simulink model "Cruise_creator"  (R2022a)
settings: Solver=FixedStepAuto  StartTime=0.0  StopTime=10  FixedStep=1e-3  RelTol=1e-3
values from neighbouring files (symbols this model uses):
  base = Mb*9.8/4+mw*9.8+0.5*9.8*Mv    (= 46305)    [parameterSetting.m]
  d = 2.6    [parameterSetting.m]
  I = 3055e-8    (= 3.055e-05)    [parameterSetting.m]
  Jv = 3044    [parameterSetting.m]
  kf = 6.67e7    (= 66700000)    [parameterSetting.m]
  Kv = 1.5e6    (= 1500000)    [parameterSetting.m]
  L = beamLength(k)*210    [BuildDataSample.m]    (also in parameterSetting.m = 210)
  Ln = length_set(i)    [DatacreatorForChapter4.m]
  Ln2 = 0    [DatacreatorForChapter4.m]
  m = 7800*(7684e-6)    (= 59.9352)    [parameterSetting.m]
  Mv = 4060/2    (= 2030)    [parameterSetting.m]
  mw = 1670/2    (= 835)    [parameterSetting.m]
  position = 0.55*L    [DatacreatorForChapter4.m]    (also in Figure4_9.m = 94.5; chapter4_3.m = 105; parameterSetting.m = 105)
  R = 0.42    [parameterSetting.m]
  r = 0.25    [chapter4_3.m]    (also in parameterSetting.m = 0.42)
  v = vkmh/3.6    [BuildDataSample.m]    (also in DatacreatorForChapter4.m = vkmh_set(j)/3.6; Figure4_9.m = vkmh_set(i); chapter4_1.m = vkmh_set(i)/3.6; chapter4_3.m = 13.8888888889)
  vkmh = vkmh_set(j)    [BuildDataSample.m]    (also in parameterSetting.m = 100)
(part 1 of 79)
settings Model: Creator=Spicydel  LastModifiedBy=Spicydel

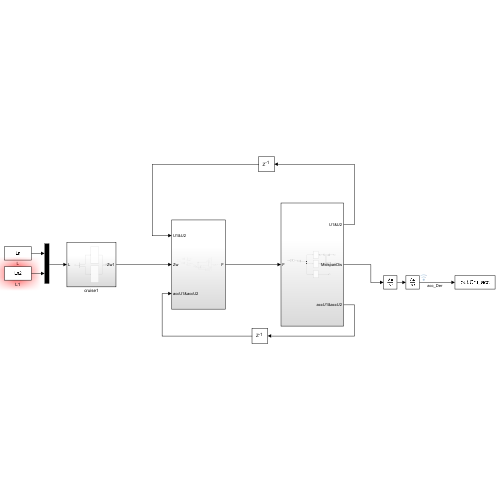
[thumbnail: Simulink's stored preview of the top-level diagram]
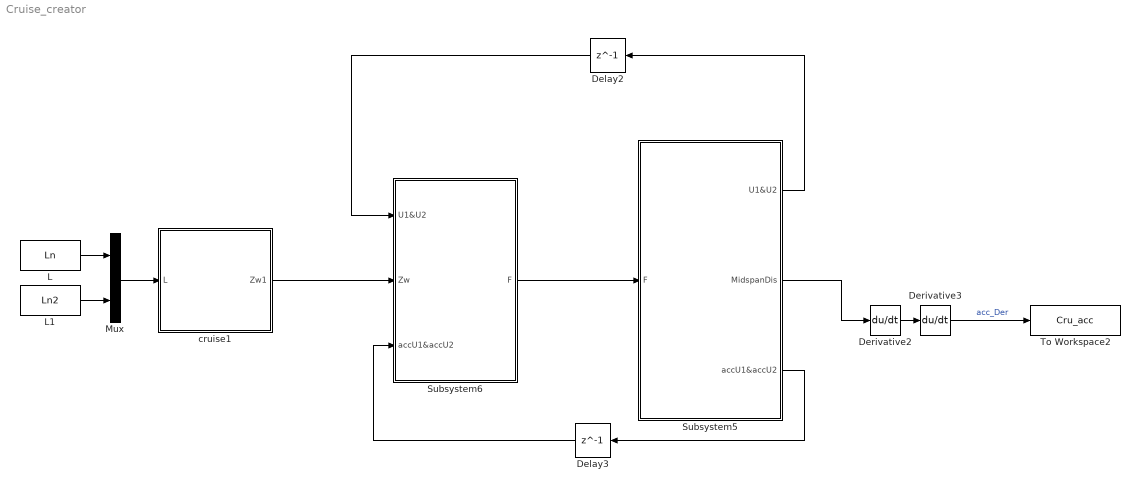
== system Cruise_creator ==
  Delay2 : Delay  DelayLength=1
  Delay3 : Delay  DelayLength=1
  Derivative2 : Derivative
  Derivative3 : Derivative
  L : Constant  Value=Ln
  L1 : Constant  Value=Ln2
  Mux : Mux  Inputs=2
  Subsystem5 : Subsystem
  Subsystem6 : Subsystem
  To Workspace2 : ToWorkspace  VariableName=Cru_acc  SaveFormat=Timeseries  SampleTime=-1
  cruise1 : Subsystem
  wires:
  Mux/1 -> cruise1/1(L)
  L1/1 -> Mux/2
  L/1 -> Mux/1
  Subsystem5/3(accU1&accU2) -> Delay3/1
  Derivative3/1 -> To Workspace2/1  "acc_Der"
  Derivative2/1 -> Derivative3/1
  Subsystem5/2(MidspanDis) -> Derivative2/1
  Delay2/1 -> Subsystem6/1(U1&U2)
  Subsystem5/1(U1&U2) -> Delay2/1
  Subsystem6/1(F) -> Subsystem5/1(F)
  cruise1/1(Zw1) -> Subsystem6/2(Zw)
  Delay3/1 -> Subsystem6/3(accU1&accU2)

== system Cruise_creator/Subsystem5 ==
  F : Inport
  Add : Sum
  Demux1 : Demux  Outputs=2
  Demux2 : Demux  Outputs=2
  Demux3 : Demux  Outputs=2
  Gain1 : Gain  Gain=-1
  Gain2 : Gain  Gain=-1
  Mux : Mux  Inputs=2
  Mux1 : Mux  Inputs=2
  Mux2 : Mux  Inputs=2
  Subsystem : Subsystem
  Subsystem1 : Subsystem
  Subsystem3 : Subsystem
  U1&U2 : Outport
  MidspanDis : Outport  Port=2
  accU1&accU2 : Outport  Port=3
  wires:
  Demux3/2 -> Mux2/2
  Demux2/2 -> Mux2/1
  Mux/1 -> U1&U2/1
  Mux2/1 -> accU1&accU2/1
  Demux3/1 -> Mux/2
  Demux2/1 -> Mux/1
  Gain2/1 -> Demux3/1
  Mux1/1 -> Subsystem/1(F), Subsystem1/1(F), Subsystem3/1(F)
  Add/1 -> Mux1/1, Mux1/2
  Demux1/2 -> Add/2
  Demux1/1 -> Add/1
  F/1 -> Demux1/1
  Gain1/1 -> Demux2/1
  Subsystem1/1(u2) -> Gain2/1
  Subsystem/1(u1) -> Gain1/1
  Subsystem3/1(Midspan) -> MidspanDis/1

== system Cruise_creator/Subsystem6 ==
  U1&U2 : Inport
  Zw : Inport  Port=2
  accU1&accU2 : Inport  Port=3
  Add : Sum  Inputs=+---
  Add1 : Sum  Inputs=-+
  Add2 : Sum  Inputs=--++-
  Add3 : Sum  Inputs=-++--
  Add4 : Sum
  Add5 : Sum  Inputs=-+
  Add6 : Sum  Inputs=-+
  Add7 : Sum  Inputs=++--
  Constant : Constant  Value=base
  Constant1 : Constant  Value=0
  Demux{,1..6} (7 alike) : Demux  Outputs=2
  Derivative{,1..3} (4 alike) : Derivative
  Gain : Gain  Gain=2*Kv/Mv
  Gain1 : Gain  Gain=Kv*d*d/Jv/2
  Gain12 : Gain  Gain=Kv/Mv
  Gain14 : Gain  Gain=Kv*d/Jv/2
  Gain2 : Gain  Gain=Kv
  Gain3 : Gain  Gain=Kv/Mv
  Gain4 : Gain  Gain=Kv*d/Jv/2
  Gain5 : Gain  Gain=d*Kv/2
  Gain7 : Gain  Gain=mw
  Gain8 : Gain  Gain=mw
  Gain9 : Gain  Gain=Kv
  Integrator{,1..3} (4 alike) : Integrator
  Max : MinMax  Function=max  Inputs=2
  Mux : Mux  Inputs=2
  F : Outport
  wires:
  Add/1 -> Mux/1  "F1"
  Add7/1 -> Mux/2  "F2"
  Gain8/1 -> Add7/4
  Demux4/2 -> Add7/3
  Gain9/1 -> Demux4/1
  Gain7/1 -> Add/4
  Add6/1 -> Gain8/1
  Add5/1 -> Gain7/1
  Demux4/1 -> Add/3
  Demux5/2 -> Add6/1
  Derivative3/1 -> Add6/2
  Demux5/1 -> Add5/1
  Derivative1/1 -> Add5/2
  Demux6/2 -> Derivative2/1
  Derivative2/1 -> Derivative3/1
  Demux6/1 -> Derivative/1
  accU1&accU2/1 -> Demux5/1
  Derivative/1 -> Derivative1/1
  Add1/1 -> Gain9/1
  [Zw] -> Add1/2, Demux6/1
  [U1u2] -> Add1/1
  [Zw] -> Gain4/1
  Gain4/1 -> Demux2/1
  Gain3/1 -> Demux/1
  [Zw] -> Gain3/1
  Gain2/1 -> Add/1, Add7/1
  Demux3/2 -> Add3/4
  Demux3/1 -> Add3/3
  Gain14/1 -> Demux3/1
  [U1u2] -> Gain14/1
  Demux1/1 -> Add2/1
  Demux1/2 -> Add2/2
  Gain12/1 -> Demux1/1
  [U1u2] -> Gain12/1
  U1&U2/1 -> [U1u2]
  Demux2/2 -> Add3/2
  Demux2/1 -> Add3/1
  Gain1/1 -> Add3/5  "theta_dot_dot"
  Demux/2 -> Add2/4
  Demux/1 -> Add2/3
  Gain/1 -> Add2/5  "Z_dot_dot"
  Zw/1 -> [Zw]
  Gain5/1 -> Add7/2, Add/2
  Add3/1 -> Integrator2/1  "theta_dot_dot"
  Integrator3/1 -> Gain1/1, Gain5/1  "theta"
  Integrator2/1 -> Integrator3/1
  Add2/1 -> Integrator/1  "Z_dot_dot"
  Integrator1/1 -> Gain/1, Gain2/1  "Zv"
  Integrator/1 -> Integrator1/1
  Constant1/1 -> Max/2
  Add4/1 -> Max/1
  Mux/1 -> Add4/1
  Constant/1 -> Add4/2
  Max/1 -> F/1

== system Cruise_creator/cruise1 ==
  L : Inport
  Demux : Demux  Outputs=2
  Mux : Mux  Inputs=2
  Subsystem1 : Subsystem
  Subsystem2 : Subsystem
  Zw1 : Outport
  wires:
  L/1 -> Demux/1
  Demux/1 -> Subsystem1/1(L0)
  Demux/2 -> Subsystem2/1(L0)
  Subsystem1/1(Output) -> Mux/1
  Subsystem2/1(Output) -> Mux/2
  Mux/1 -> Zw1/1

== system Cruise_creator/Subsystem5/Subsystem ==
  F : Inport
  Add : Sum  Inputs=+++++++
  Constant1 : Constant  Value=21
  Constant11 : Constant
  Constant2 : Constant  Value=41
  Constant3 : Constant  Value=61
  Constant4 : Constant  Value=81
  Constant5 : Constant  Value=101
  Constant6 : Constant  Value=121
  Subsystem : Subsystem
  Subsystem1 : Subsystem
  Subsystem2 : Subsystem
  Subsystem3 : Subsystem
  Subsystem4 : Subsystem
  Subsystem5 : Subsystem
  Subsystem6 : Subsystem
  u1 : Outport
  wires:
  Subsystem6/1(Out1) -> Add/7
  [Ff] -> Subsystem6/2(In2)
  Constant6/1 -> Subsystem6/1(In1)
  Subsystem5/1(Out1) -> Add/6
  Constant5/1 -> Subsystem5/1(In1)
  [Ff] -> Subsystem5/2(In2)
  Add/1 -> u1/1
  Subsystem4/1(Out1) -> Add/5
  Subsystem3/1(Out1) -> Add/4
  Subsystem2/1(Out1) -> Add/3
  Subsystem1/1(Out1) -> Add/2
  Subsystem/1(Out1) -> Add/1
  [Ff] -> Subsystem4/2(In2)
  Constant4/1 -> Subsystem4/1(In1)
  Constant3/1 -> Subsystem3/1(In1)
  [Ff] -> Subsystem3/2(In2)
  [Ff] -> Subsystem2/2(In2)
  Constant2/1 -> Subsystem2/1(In1)
  Constant1/1 -> Subsystem1/1(In1)
  [Ff] -> Subsystem1/2(In2)
  [Ff] -> Subsystem/2(In2)
  Constant11/1 -> Subsystem/1(In1)
  F/1 -> [Ff]

== system Cruise_creator/Subsystem5/Subsystem1 ==
  F : Inport
  Add : Sum  Inputs=+++++++
  Constant1 : Constant  Value=21
  Constant11 : Constant
  Constant2 : Constant  Value=61
  Constant3 : Constant  Value=41
  Constant4 : Constant  Value=101
  Constant5 : Constant  Value=81
  Constant6 : Constant  Value=121
  Subsystem : Subsystem
  Subsystem1 : Subsystem
  Subsystem2 : Subsystem
  Subsystem3 : Subsystem
  Subsystem4 : Subsystem
  Subsystem5 : Subsystem
  Subsystem6 : Subsystem
  u2 : Outport
  wires:
  Subsystem6/1(Out1) -> Add/7
  Subsystem5/1(Out1) -> Add/6
  Constant4/1 -> Subsystem5/1(In1)
  [Ff] -> Subsystem5/2(In2)
  Constant6/1 -> Subsystem6/1(In1)
  [Ff] -> Subsystem6/2(In2)
  Add/1 -> u2/1
  Subsystem4/1(Out1) -> Add/5
  Subsystem3/1(Out1) -> Add/4
  Subsystem2/1(Out1) -> Add/3
  Subsystem1/1(Out1) -> Add/2
  [Ff] -> Subsystem4/2(In2)
  Constant5/1 -> Subsystem4/1(In1)
  Constant3/1 -> Subsystem2/1(In1)
  [Ff] -> Subsystem2/2(In2)
  [Ff] -> Subsystem3/2(In2)
  Constant2/1 -> Subsystem3/1(In1)
  Constant1/1 -> Subsystem1/1(In1)
  [Ff] -> Subsystem1/2(In2)
  [Ff] -> Subsystem/2(In2)
  Subsystem/1(Out1) -> Add/1
  Constant11/1 -> Subsystem/1(In1)
  F/1 -> [Ff]

== system Cruise_creator/Subsystem5/Subsystem3 ==
  F : Inport
  Add : Sum  Inputs=+++++++
  Constant1 : Constant  Value=21
  Constant11 : Constant
  Constant2 : Constant  Value=41
  Constant3 : Constant  Value=61
  Constant4 : Constant  Value=81
  Constant5 : Constant  Value=101
  Constant6 : Constant  Value=121
  Subsystem1 : Subsystem
  Subsystem2 : Subsystem
  Subsystem3 : Subsystem
  Subsystem4 : Subsystem
  Subsystem5 : Subsystem
  Subsystem6 : Subsystem
  Subsystem7 : Subsystem
  Midspan : Outport
  wires:
  Subsystem7/1(Out1) -> Add/7
  Subsystem6/1(Out1) -> Add/6
  [Ff] -> Subsystem7/1(In2)
  Constant6/1 -> Subsystem7/2(In1)
  Constant5/1 -> Subsystem6/2(In1)
  [Ff] -> Subsystem6/1(In2)
  Subsystem4/1(Out1) -> Add/5
  Subsystem3/1(Out1) -> Add/4
  [Ff] -> Subsystem4/1(In2)
  Constant4/1 -> Subsystem4/2(In1)
  Constant3/1 -> Subsystem3/2(In1)
  [Ff] -> Subsystem3/1(In2)
  [Ff] -> Subsystem2/1(In2)
  [Ff] -> Subsystem1/1(In2)
  [Ff] -> Subsystem5/1(In2)
  Add/1 -> Midspan/1
  Subsystem2/1(Out1) -> Add/3
  Constant2/1 -> Subsystem2/2(In1)
  Subsystem1/1(Out1) -> Add/2
  Constant1/1 -> Subsystem1/2(In1)
  Subsystem5/1(Out1) -> Add/1
  Constant11/1 -> Subsystem5/2(In1)
  F/1 -> [Ff]

== system Cruise_creator/cruise1/Subsystem1 ==
  L0 : Inport
  Constant : Constant  Value=0
  Scope : Scope
  Subsystem : Subsystem
  Switch : Switch  Criteria=u2 ~= 0  SaturateOnIntegerOverflow=off  InputSameDT=off
  Output : Outport
  wires:
  L0/1 -> Subsystem/1(L), Subsystem/2(L0), Switch/2
  Switch/1 -> Output/1
  Constant/1 -> Switch/3
  Subsystem/1(Output) -> Scope/1, Switch/1

== system Cruise_creator/cruise1/Subsystem2 ==
  L0 : Inport
  Constant : Constant  Value=0
  Subsystem : Subsystem
  Switch : Switch  Criteria=u2 ~= 0  SaturateOnIntegerOverflow=off  InputSameDT=off
  Output : Outport
  wires:
  Subsystem/1(Output) -> Switch/1
  Constant/1 -> Switch/3
  Switch/1 -> Output/1
  L0/1 -> Subsystem/2(L0), Switch/2, Subsystem/1(L)

== system Cruise_creator/Subsystem5/Subsystem/Subsystem ==
  In1 : Inport
  In2 : Inport  Port=2
  Add : Sum  Inputs=++++++++++++++++++++
  Add Constant{,1..18} (19 alike) : Bias  Bias=1  SaturateOnIntegerOverflow=off
  Subsystem1 : Subsystem
  Subsystem10 : Subsystem
  Subsystem11 : Subsystem
  Subsystem12 : Subsystem
  Subsystem13 : Subsystem
  Subsystem14 : Subsystem
  Subsystem15 : Subsystem
  Subsystem16 : Subsystem
  Subsystem17 : Subsystem
  Subsystem18 : Subsystem
  Subsystem19 : Subsystem
  Subsystem2 : Subsystem
  Subsystem20 : Subsystem
  Subsystem3 : Subsystem
  Subsystem4 : Subsystem
  Subsystem5 : Subsystem
  Subsystem6 : Subsystem
  Subsystem7 : Subsystem
  Subsystem8 : Subsystem
  Subsystem9 : Subsystem
  Out1 : Outport
  wires:
  In2/1 -> [Ff]
  Add/1 -> Out1/1
  [Ff] -> Subsystem18/2(F)
  [Ff] -> Subsystem16/2(F)
  Add Constant7/1 -> Add Constant8/1, Subsystem14/1(index)
  Add Constant4/1 -> Add Constant5/1, Subsystem4/1(index)
  Add Constant6/1 -> Add Constant7/1, Subsystem5/1(index)
  Add Constant5/1 -> Add Constant6/1, Subsystem7/1(index)
  Subsystem11/1(Out1) -> Add/11
  Subsystem18/1(Out1) -> Add/20
  [Ff] -> Subsystem12/2(F)
  [Ff] -> Subsystem14/2(F)
  [Ff] -> Subsystem5/2(F)
  [Ff] -> Subsystem9/2(F)
  [Ff] -> Subsystem4/2(F)
  Subsystem4/1(Out1) -> Add/6
  Add Constant12/1 -> Add Constant13/1, Subsystem12/1(index)
  Subsystem2/1(Out1) -> Add/4
  Add Constant13/1 -> Add Constant14/1, Subsystem15/1(index)
  Subsystem15/1(Out1) -> Add/15
  [Ff] -> Subsystem11/2(F)
  [Ff] -> Subsystem7/2(F)
  [Ff] -> Subsystem6/2(F)
  Subsystem13/1(Out1) -> Add/16
  Subsystem16/1(Out1) -> Add/13
  In1/1 -> Add Constant/1, Subsystem6/1(index)
  Subsystem12/1(Out1) -> Add/14
  Subsystem20/1(Out1) -> Add/17
  [Ff] -> Subsystem8/2(F)
  Subsystem3/1(Out1) -> Add/3
  [Ff] -> Subsystem2/2(F)
  Add Constant8/1 -> Add Constant9/1, Subsystem9/1(index)
  [Ff] -> Subsystem19/2(F)
  [Ff] -> Subsystem1/2(F)
  Subsystem19/1(Out1) -> Add/19
  Subsystem17/1(Out1) -> Add/18
  [Ff] -> Subsystem20/2(F)
  Add Constant10/1 -> Add Constant11/1, Subsystem10/1(index)
  Add Constant16/1 -> Add Constant17/1, Subsystem17/1(index)
  Subsystem6/1(Out1) -> Add/1
  Subsystem10/1(Out1) -> Add/12
  Subsystem5/1(Out1) -> Add/8
  [Ff] -> Subsystem10/2(F)
  [Ff] -> Subsystem15/2(F)
  Add Constant18/1 -> Subsystem18/1(index)
  Add Constant17/1 -> Add Constant18/1, Subsystem19/1(index)
  Subsystem7/1(Out1) -> Add/7
  Add Constant1/1 -> Add Constant2/1, Subsystem3/1(index)
  [Ff] -> Subsystem3/2(F)
  Subsystem9/1(Out1) -> Add/10
  Add Constant3/1 -> Add Constant4/1, Subsystem8/1(index)
  Add Constant/1 -> Add Constant1/1, Subsystem1/1(index)
  Subsystem1/1(Out1) -> Add/2
  Add Constant15/1 -> Add Constant16/1, Subsystem20/1(index)
  [Ff] -> Subsystem17/2(F)
  Subsystem8/1(Out1) -> Add/5
  Add Constant14/1 -> Add Constant15/1, Subsystem13/1(index)
  Add Constant11/1 -> Add Constant12/1, Subsystem16/1(index)
  [Ff] -> Subsystem13/2(F)
  Add Constant2/1 -> Add Constant3/1, Subsystem2/1(index)
  Add Constant9/1 -> Add Constant10/1, Subsystem11/1(index)
  Subsystem14/1(Out1) -> Add/9

== system Cruise_creator/Subsystem5/Subsystem/Subsystem1 ==
  In1 : Inport
  In2 : Inport  Port=2
  Add : Sum  Inputs=++++++++++++++++++++
  Add Constant{,1..18} (19 alike) : Bias  Bias=1  SaturateOnIntegerOverflow=off
  Subsystem1 : Subsystem
  Subsystem10 : Subsystem
  Subsystem11 : Subsystem
  Subsystem12 : Subsystem
  Subsystem13 : Subsystem
  Subsystem14 : Subsystem
  Subsystem15 : Subsystem
  Subsystem16 : Subsystem
  Subsystem17 : Subsystem
  Subsystem18 : Subsystem
  Subsystem19 : Subsystem
  Subsystem2 : Subsystem
  Subsystem20 : Subsystem
  Subsystem3 : Subsystem
  Subsystem4 : Subsystem
  Subsystem5 : Subsystem
  Subsystem6 : Subsystem
  Subsystem7 : Subsystem
  Subsystem8 : Subsystem
  Subsystem9 : Subsystem
  Out1 : Outport
  wires:
  Subsystem14/1(Out1) -> Add/9
  Add Constant9/1 -> Subsystem11/1(index), Add Constant10/1
  Add Constant2/1 -> Subsystem2/1(index), Add Constant3/1
  [Ff] -> Subsystem13/2(F)
  Add Constant11/1 -> Subsystem16/1(index), Add Constant12/1
  Add Constant14/1 -> Subsystem13/1(index), Add Constant15/1
  Subsystem8/1(Out1) -> Add/5
  [Ff] -> Subsystem17/2(F)
  Add Constant15/1 -> Subsystem20/1(index), Add Constant16/1
  Subsystem1/1(Out1) -> Add/2
  Add Constant/1 -> Subsystem1/1(index), Add Constant1/1
  Add Constant3/1 -> Subsystem8/1(index), Add Constant4/1
  Subsystem9/1(Out1) -> Add/10
  [Ff] -> Subsystem3/2(F)
  Add Constant1/1 -> Subsystem3/1(index), Add Constant2/1
  Subsystem7/1(Out1) -> Add/7
  Add Constant17/1 -> Subsystem19/1(index), Add Constant18/1
  Add Constant18/1 -> Subsystem18/1(index)
  [Ff] -> Subsystem15/2(F)
  [Ff] -> Subsystem10/2(F)
  Subsystem5/1(Out1) -> Add/8
  Subsystem10/1(Out1) -> Add/12
  Subsystem6/1(Out1) -> Add/1
  Add Constant16/1 -> Subsystem17/1(index), Add Constant17/1
  Add Constant10/1 -> Subsystem10/1(index), Add Constant11/1
  [Ff] -> Subsystem20/2(F)
  Subsystem17/1(Out1) -> Add/18
  Subsystem19/1(Out1) -> Add/19
  [Ff] -> Subsystem1/2(F)
  [Ff] -> Subsystem19/2(F)
  Add Constant8/1 -> Subsystem9/1(index), Add Constant9/1
  [Ff] -> Subsystem2/2(F)
  Subsystem3/1(Out1) -> Add/3
  [Ff] -> Subsystem8/2(F)
  Subsystem20/1(Out1) -> Add/17
  Subsystem12/1(Out1) -> Add/14
  In1/1 -> Subsystem6/1(index), Add Constant/1
  Subsystem16/1(Out1) -> Add/13
  Subsystem13/1(Out1) -> Add/16
  [Ff] -> Subsystem6/2(F)
  [Ff] -> Subsystem7/2(F)
  [Ff] -> Subsystem11/2(F)
  Subsystem15/1(Out1) -> Add/15
  Add Constant13/1 -> Subsystem15/1(index), Add Constant14/1
  Subsystem2/1(Out1) -> Add/4
  Add Constant12/1 -> Subsystem12/1(index), Add Constant13/1
  Subsystem4/1(Out1) -> Add/6
  [Ff] -> Subsystem4/2(F)
  [Ff] -> Subsystem9/2(F)
  [Ff] -> Subsystem5/2(F)
  [Ff] -> Subsystem14/2(F)
  [Ff] -> Subsystem12/2(F)
  Subsystem18/1(Out1) -> Add/20
  Subsystem11/1(Out1) -> Add/11
  Add Constant5/1 -> Subsystem7/1(index), Add Constant6/1
  Add Constant6/1 -> Subsystem5/1(index), Add Constant7/1
  Add Constant4/1 -> Subsystem4/1(index), Add Constant5/1
  Add Constant7/1 -> Subsystem14/1(index), Add Constant8/1
  [Ff] -> Subsystem16/2(F)
  [Ff] -> Subsystem18/2(F)
  Add/1 -> Out1/1
  In2/1 -> [Ff]

== system Cruise_creator/Subsystem5/Subsystem/Subsystem2 ==
  In1 : Inport
  In2 : Inport  Port=2
  Add : Sum  Inputs=++++++++++++++++++++
  Add Constant{,1..18} (19 alike) : Bias  Bias=1  SaturateOnIntegerOverflow=off
  Subsystem1 : Subsystem
  Subsystem10 : Subsystem
  Subsystem11 : Subsystem
  Subsystem12 : Subsystem
  Subsystem13 : Subsystem
  Subsystem14 : Subsystem
  Subsystem15 : Subsystem
  Subsystem16 : Subsystem
  Subsystem17 : Subsystem
  Subsystem18 : Subsystem
  Subsystem19 : Subsystem
  Subsystem2 : Subsystem
  Subsystem20 : Subsystem
  Subsystem3 : Subsystem
  Subsystem4 : Subsystem
  Subsystem5 : Subsystem
  Subsystem6 : Subsystem
  Subsystem7 : Subsystem
  Subsystem8 : Subsystem
  Subsystem9 : Subsystem
  Out1 : Outport
  wires:
  In2/1 -> [Ff]
  Add/1 -> Out1/1
  [Ff] -> Subsystem18/2(F)
  [Ff] -> Subsystem16/2(F)
  Add Constant7/1 -> Add Constant8/1, Subsystem14/1(index)
  Add Constant4/1 -> Add Constant5/1, Subsystem4/1(index)
  Add Constant6/1 -> Add Constant7/1, Subsystem5/1(index)
  Add Constant5/1 -> Add Constant6/1, Subsystem7/1(index)
  Subsystem11/1(Out1) -> Add/11
  Subsystem18/1(Out1) -> Add/20
  [Ff] -> Subsystem12/2(F)
  [Ff] -> Subsystem14/2(F)
  [Ff] -> Subsystem5/2(F)
  [Ff] -> Subsystem9/2(F)
  [Ff] -> Subsystem4/2(F)
  Subsystem4/1(Out1) -> Add/6
  Add Constant12/1 -> Add Constant13/1, Subsystem12/1(index)
  Subsystem2/1(Out1) -> Add/4
  Add Constant13/1 -> Add Constant14/1, Subsystem15/1(index)
  Subsystem15/1(Out1) -> Add/15
  [Ff] -> Subsystem11/2(F)
  [Ff] -> Subsystem7/2(F)
  [Ff] -> Subsystem6/2(F)
  Subsystem13/1(Out1) -> Add/16
  Subsystem16/1(Out1) -> Add/13
  In1/1 -> Add Constant/1, Subsystem6/1(index)
  Subsystem12/1(Out1) -> Add/14
  Subsystem20/1(Out1) -> Add/17
  [Ff] -> Subsystem8/2(F)
  Subsystem3/1(Out1) -> Add/3
  [Ff] -> Subsystem2/2(F)
  Add Constant8/1 -> Add Constant9/1, Subsystem9/1(index)
  [Ff] -> Subsystem19/2(F)
  [Ff] -> Subsystem1/2(F)
  Subsystem19/1(Out1) -> Add/19
  Subsystem17/1(Out1) -> Add/18
  [Ff] -> Subsystem20/2(F)
  Add Constant10/1 -> Add Constant11/1, Subsystem10/1(index)
  Add Constant16/1 -> Add Constant17/1, Subsystem17/1(index)
  Subsystem6/1(Out1) -> Add/1
  Subsystem10/1(Out1) -> Add/12
  Subsystem5/1(Out1) -> Add/8
  [Ff] -> Subsystem10/2(F)
  [Ff] -> Subsystem15/2(F)
  Add Constant18/1 -> Subsystem18/1(index)
  Add Constant17/1 -> Add Constant18/1, Subsystem19/1(index)
  Subsystem7/1(Out1) -> Add/7
  Add Constant1/1 -> Add Constant2/1, Subsystem3/1(index)
  [Ff] -> Subsystem3/2(F)
  Subsystem9/1(Out1) -> Add/10
  Add Constant3/1 -> Add Constant4/1, Subsystem8/1(index)
  Add Constant/1 -> Add Constant1/1, Subsystem1/1(index)
  Subsystem1/1(Out1) -> Add/2
  Add Constant15/1 -> Add Constant16/1, Subsystem20/1(index)
  [Ff] -> Subsystem17/2(F)
  Subsystem8/1(Out1) -> Add/5
  Add Constant14/1 -> Add Constant15/1, Subsystem13/1(index)
  Add Constant11/1 -> Add Constant12/1, Subsystem16/1(index)
  [Ff] -> Subsystem13/2(F)
  Add Constant2/1 -> Add Constant3/1, Subsystem2/1(index)
  Add Constant9/1 -> Add Constant10/1, Subsystem11/1(index)
  Subsystem14/1(Out1) -> Add/9

== system Cruise_creator/Subsystem5/Subsystem/Subsystem3 ==
  In1 : Inport
  In2 : Inport  Port=2
  Add : Sum  Inputs=++++++++++++++++++++
  Add Constant{,1..18} (19 alike) : Bias  Bias=1  SaturateOnIntegerOverflow=off
  Subsystem1 : Subsystem
  Subsystem10 : Subsystem
  Subsystem11 : Subsystem
  Subsystem12 : Subsystem
  Subsystem13 : Subsystem
  Subsystem14 : Subsystem
  Subsystem15 : Subsystem
  Subsystem16 : Subsystem
  Subsystem17 : Subsystem
  Subsystem18 : Subsystem
  Subsystem19 : Subsystem
  Subsystem2 : Subsystem
  Subsystem20 : Subsystem
  Subsystem3 : Subsystem
  Subsystem4 : Subsystem
  Subsystem5 : Subsystem
  Subsystem6 : Subsystem
  Subsystem7 : Subsystem
  Subsystem8 : Subsystem
  Subsystem9 : Subsystem
  Out1 : Outport
  wires:
  Subsystem14/1(Out1) -> Add/9
  Add Constant9/1 -> Subsystem11/1(index), Add Constant10/1
  Add Constant2/1 -> Subsystem2/1(index), Add Constant3/1
  [Ff] -> Subsystem13/2(F)
  Add Constant11/1 -> Subsystem16/1(index), Add Constant12/1
  Add Constant14/1 -> Subsystem13/1(index), Add Constant15/1
  Subsystem8/1(Out1) -> Add/5
  [Ff] -> Subsystem17/2(F)
  Add Constant15/1 -> Subsystem20/1(index), Add Constant16/1
  Subsystem1/1(Out1) -> Add/2
  Add Constant/1 -> Subsystem1/1(index), Add Constant1/1
  Add Constant3/1 -> Subsystem8/1(index), Add Constant4/1
  Subsystem9/1(Out1) -> Add/10
  [Ff] -> Subsystem3/2(F)
  Add Constant1/1 -> Subsystem3/1(index), Add Constant2/1
  Subsystem7/1(Out1) -> Add/7
  Add Constant17/1 -> Subsystem19/1(index), Add Constant18/1
  Add Constant18/1 -> Subsystem18/1(index)
  [Ff] -> Subsystem15/2(F)
  [Ff] -> Subsystem10/2(F)
  Subsystem5/1(Out1) -> Add/8
  Subsystem10/1(Out1) -> Add/12
  Subsystem6/1(Out1) -> Add/1
  Add Constant16/1 -> Subsystem17/1(index), Add Constant17/1
  Add Constant10/1 -> Subsystem10/1(index), Add Constant11/1
  [Ff] -> Subsystem20/2(F)
  Subsystem17/1(Out1) -> Add/18
  Subsystem19/1(Out1) -> Add/19
  [Ff] -> Subsystem1/2(F)
  [Ff] -> Subsystem19/2(F)
  Add Constant8/1 -> Subsystem9/1(index), Add Constant9/1
  [Ff] -> Subsystem2/2(F)
  Subsystem3/1(Out1) -> Add/3
  [Ff] -> Subsystem8/2(F)
  Subsystem20/1(Out1) -> Add/17
  Subsystem12/1(Out1) -> Add/14
  In1/1 -> Subsystem6/1(index), Add Constant/1
  Subsystem16/1(Out1) -> Add/13
  Subsystem13/1(Out1) -> Add/16
  [Ff] -> Subsystem6/2(F)
  [Ff] -> Subsystem7/2(F)
  [Ff] -> Subsystem11/2(F)
  Subsystem15/1(Out1) -> Add/15
  Add Constant13/1 -> Subsystem15/1(index), Add Constant14/1
  Subsystem2/1(Out1) -> Add/4
  Add Constant12/1 -> Subsystem12/1(index), Add Constant13/1
  Subsystem4/1(Out1) -> Add/6
  [Ff] -> Subsystem4/2(F)
  [Ff] -> Subsystem9/2(F)
  [Ff] -> Subsystem5/2(F)
  [Ff] -> Subsystem14/2(F)
  [Ff] -> Subsystem12/2(F)
  Subsystem18/1(Out1) -> Add/20
  Subsystem11/1(Out1) -> Add/11
  Add Constant5/1 -> Subsystem7/1(index), Add Constant6/1
  Add Constant6/1 -> Subsystem5/1(index), Add Constant7/1
  Add Constant4/1 -> Subsystem4/1(index), Add Constant5/1
  Add Constant7/1 -> Subsystem14/1(index), Add Constant8/1
  [Ff] -> Subsystem16/2(F)
  [Ff] -> Subsystem18/2(F)
  Add/1 -> Out1/1
  In2/1 -> [Ff]

== system Cruise_creator/Subsystem5/Subsystem/Subsystem4 ==
  In1 : Inport
  In2 : Inport  Port=2
  Add : Sum  Inputs=++++++++++++++++++++
  Add Constant{,1..18} (19 alike) : Bias  Bias=1  SaturateOnIntegerOverflow=off
  Subsystem1 : Subsystem
  Subsystem10 : Subsystem
  Subsystem11 : Subsystem
  Subsystem12 : Subsystem
  Subsystem13 : Subsystem
  Subsystem14 : Subsystem
  Subsystem15 : Subsystem
  Subsystem16 : Subsystem
  Subsystem17 : Subsystem
  Subsystem18 : Subsystem
  Subsystem19 : Subsystem
  Subsystem2 : Subsystem
  Subsystem20 : Subsystem
  Subsystem3 : Subsystem
  Subsystem4 : Subsystem
  Subsystem5 : Subsystem
  Subsystem6 : Subsystem
  Subsystem7 : Subsystem
  Subsystem8 : Subsystem
  Subsystem9 : Subsystem
  Out1 : Outport
  wires:
  In2/1 -> [Ff]
  Add/1 -> Out1/1
  [Ff] -> Subsystem18/2(F)
  [Ff] -> Subsystem16/2(F)
  Add Constant7/1 -> Add Constant8/1, Subsystem14/1(index)
  Add Constant4/1 -> Add Constant5/1, Subsystem4/1(index)
  Add Constant6/1 -> Add Constant7/1, Subsystem5/1(index)
  Add Constant5/1 -> Add Constant6/1, Subsystem7/1(index)
  Subsystem11/1(Out1) -> Add/11
  Subsystem18/1(Out1) -> Add/20
  [Ff] -> Subsystem12/2(F)
  [Ff] -> Subsystem14/2(F)
  [Ff] -> Subsystem5/2(F)
  [Ff] -> Subsystem9/2(F)
  [Ff] -> Subsystem4/2(F)
  Subsystem4/1(Out1) -> Add/6
  Add Constant12/1 -> Add Constant13/1, Subsystem12/1(index)
  Subsystem2/1(Out1) -> Add/4
  Add Constant13/1 -> Add Constant14/1, Subsystem15/1(index)
  Subsystem15/1(Out1) -> Add/15
  [Ff] -> Subsystem11/2(F)
  [Ff] -> Subsystem7/2(F)
  [Ff] -> Subsystem6/2(F)
  Subsystem13/1(Out1) -> Add/16
  Subsystem16/1(Out1) -> Add/13
  In1/1 -> Add Constant/1, Subsystem6/1(index)
  Subsystem12/1(Out1) -> Add/14
  Subsystem20/1(Out1) -> Add/17
  [Ff] -> Subsystem8/2(F)
  Subsystem3/1(Out1) -> Add/3
  [Ff] -> Subsystem2/2(F)
  Add Constant8/1 -> Add Constant9/1, Subsystem9/1(index)
  [Ff] -> Subsystem19/2(F)
  [Ff] -> Subsystem1/2(F)
  Subsystem19/1(Out1) -> Add/19
  Subsystem17/1(Out1) -> Add/18
  [Ff] -> Subsystem20/2(F)
  Add Constant10/1 -> Add Constant11/1, Subsystem10/1(index)
  Add Constant16/1 -> Add Constant17/1, Subsystem17/1(index)
  Subsystem6/1(Out1) -> Add/1
  Subsystem10/1(Out1) -> Add/12
  Subsystem5/1(Out1) -> Add/8
  [Ff] -> Subsystem10/2(F)
  [Ff] -> Subsystem15/2(F)
  Add Constant18/1 -> Subsystem18/1(index)
  Add Constant17/1 -> Add Constant18/1, Subsystem19/1(index)
  Subsystem7/1(Out1) -> Add/7
  Add Constant1/1 -> Add Constant2/1, Subsystem3/1(index)
  [Ff] -> Subsystem3/2(F)
  Subsystem9/1(Out1) -> Add/10
  Add Constant3/1 -> Add Constant4/1, Subsystem8/1(index)
  Add Constant/1 -> Add Constant1/1, Subsystem1/1(index)
  Subsystem1/1(Out1) -> Add/2
  Add Constant15/1 -> Add Constant16/1, Subsystem20/1(index)
  [Ff] -> Subsystem17/2(F)
  Subsystem8/1(Out1) -> Add/5
  Add Constant14/1 -> Add Constant15/1, Subsystem13/1(index)
  Add Constant11/1 -> Add Constant12/1, Subsystem16/1(index)
  [Ff] -> Subsystem13/2(F)
  Add Constant2/1 -> Add Constant3/1, Subsystem2/1(index)
  Add Constant9/1 -> Add Constant10/1, Subsystem11/1(index)
  Subsystem14/1(Out1) -> Add/9

== system Cruise_creator/Subsystem5/Subsystem/Subsystem5 ==
  In1 : Inport
  In2 : Inport  Port=2
  Add : Sum  Inputs=++++++++++++++++++++
  Add Constant{,1..18} (19 alike) : Bias  Bias=1  SaturateOnIntegerOverflow=off
  Subsystem1 : Subsystem
  Subsystem10 : Subsystem
  Subsystem11 : Subsystem
  Subsystem12 : Subsystem
  Subsystem13 : Subsystem
  Subsystem14 : Subsystem
  Subsystem15 : Subsystem
  Subsystem16 : Subsystem
  Subsystem17 : Subsystem
  Subsystem18 : Subsystem
  Subsystem19 : Subsystem
  Subsystem2 : Subsystem
  Subsystem20 : Subsystem
  Subsystem3 : Subsystem
  Subsystem4 : Subsystem
  Subsystem5 : Subsystem
  Subsystem6 : Subsystem
  Subsystem7 : Subsystem
  Subsystem8 : Subsystem
  Subsystem9 : Subsystem
  Out1 : Outport
  wires:
  Subsystem14/1(Out1) -> Add/9
  Add Constant9/1 -> Subsystem11/1(index), Add Constant10/1
  Add Constant2/1 -> Subsystem2/1(index), Add Constant3/1
  [Ff] -> Subsystem13/2(F)
  Add Constant11/1 -> Subsystem16/1(index), Add Constant12/1
  Add Constant14/1 -> Subsystem13/1(index), Add Constant15/1
  Subsystem8/1(Out1) -> Add/5
  [Ff] -> Subsystem17/2(F)
  Add Constant15/1 -> Subsystem20/1(index), Add Constant16/1
  Subsystem1/1(Out1) -> Add/2
  Add Constant/1 -> Subsystem1/1(index), Add Constant1/1
  Add Constant3/1 -> Subsystem8/1(index), Add Constant4/1
  Subsystem9/1(Out1) -> Add/10
  [Ff] -> Subsystem3/2(F)
  Add Constant1/1 -> Subsystem3/1(index), Add Constant2/1
  Subsystem7/1(Out1) -> Add/7
  Add Constant17/1 -> Subsystem19/1(index), Add Constant18/1
  Add Constant18/1 -> Subsystem18/1(index)
  [Ff] -> Subsystem15/2(F)
  [Ff] -> Subsystem10/2(F)
  Subsystem5/1(Out1) -> Add/8
  Subsystem10/1(Out1) -> Add/12
  Subsystem6/1(Out1) -> Add/1
  Add Constant16/1 -> Subsystem17/1(index), Add Constant17/1
  Add Constant10/1 -> Subsystem10/1(index), Add Constant11/1
  [Ff] -> Subsystem20/2(F)
  Subsystem17/1(Out1) -> Add/18
  Subsystem19/1(Out1) -> Add/19
  [Ff] -> Subsystem1/2(F)
  [Ff] -> Subsystem19/2(F)
  Add Constant8/1 -> Subsystem9/1(index), Add Constant9/1
  [Ff] -> Subsystem2/2(F)
  Subsystem3/1(Out1) -> Add/3
  [Ff] -> Subsystem8/2(F)
  Subsystem20/1(Out1) -> Add/17
  Subsystem12/1(Out1) -> Add/14
  In1/1 -> Subsystem6/1(index), Add Constant/1
  Subsystem16/1(Out1) -> Add/13
  Subsystem13/1(Out1) -> Add/16
  [Ff] -> Subsystem6/2(F)
  [Ff] -> Subsystem7/2(F)
  [Ff] -> Subsystem11/2(F)
  Subsystem15/1(Out1) -> Add/15
  Add Constant13/1 -> Subsystem15/1(index), Add Constant14/1
  Subsystem2/1(Out1) -> Add/4
  Add Constant12/1 -> Subsystem12/1(index), Add Constant13/1
  Subsystem4/1(Out1) -> Add/6
  [Ff] -> Subsystem4/2(F)
  [Ff] -> Subsystem9/2(F)
  [Ff] -> Subsystem5/2(F)
  [Ff] -> Subsystem14/2(F)
  [Ff] -> Subsystem12/2(F)
  Subsystem18/1(Out1) -> Add/20
  Subsystem11/1(Out1) -> Add/11
  Add Constant5/1 -> Subsystem7/1(index), Add Constant6/1
  Add Constant6/1 -> Subsystem5/1(index), Add Constant7/1
  Add Constant4/1 -> Subsystem4/1(index), Add Constant5/1
  Add Constant7/1 -> Subsystem14/1(index), Add Constant8/1
  [Ff] -> Subsystem16/2(F)
  [Ff] -> Subsystem18/2(F)
  Add/1 -> Out1/1
  In2/1 -> [Ff]

== system Cruise_creator/Subsystem5/Subsystem/Subsystem6 ==
  In1 : Inport
  In2 : Inport  Port=2
  Add : Sum  Inputs=++++++++++++++++++++
  Add Constant{,1..18} (19 alike) : Bias  Bias=1  SaturateOnIntegerOverflow=off
  Subsystem1 : Subsystem
  Subsystem10 : Subsystem
  Subsystem11 : Subsystem
  Subsystem12 : Subsystem
  Subsystem13 : Subsystem
  Subsystem14 : Subsystem
  Subsystem15 : Subsystem
  Subsystem16 : Subsystem
  Subsystem17 : Subsystem
  Subsystem18 : Subsystem
  Subsystem19 : Subsystem
  Subsystem2 : Subsystem
  Subsystem20 : Subsystem
  Subsystem3 : Subsystem
  Subsystem4 : Subsystem
  Subsystem5 : Subsystem
  Subsystem6 : Subsystem
  Subsystem7 : Subsystem
  Subsystem8 : Subsystem
  Subsystem9 : Subsystem
  Out1 : Outport
  wires:
  In2/1 -> [Ff]
  Add/1 -> Out1/1
  [Ff] -> Subsystem18/2(F)
  [Ff] -> Subsystem16/2(F)
  Add Constant7/1 -> Add Constant8/1, Subsystem14/1(index)
  Add Constant4/1 -> Add Constant5/1, Subsystem4/1(index)
  Add Constant6/1 -> Add Constant7/1, Subsystem5/1(index)
  Add Constant5/1 -> Add Constant6/1, Subsystem7/1(index)
  Subsystem11/1(Out1) -> Add/11
  Subsystem18/1(Out1) -> Add/20
  [Ff] -> Subsystem12/2(F)
  [Ff] -> Subsystem14/2(F)
  [Ff] -> Subsystem5/2(F)
  [Ff] -> Subsystem9/2(F)
  [Ff] -> Subsystem4/2(F)
  Subsystem4/1(Out1) -> Add/6
  Add Constant12/1 -> Add Constant13/1, Subsystem12/1(index)
  Subsystem2/1(Out1) -> Add/4
  Add Constant13/1 -> Add Constant14/1, Subsystem15/1(index)
  Subsystem15/1(Out1) -> Add/15
  [Ff] -> Subsystem11/2(F)
  [Ff] -> Subsystem7/2(F)
  [Ff] -> Subsystem6/2(F)
  Subsystem13/1(Out1) -> Add/16
  Subsystem16/1(Out1) -> Add/13
  In1/1 -> Add Constant/1, Subsystem6/1(index)
  Subsystem12/1(Out1) -> Add/14
  Subsystem20/1(Out1) -> Add/17
  [Ff] -> Subsystem8/2(F)
  Subsystem3/1(Out1) -> Add/3
  [Ff] -> Subsystem2/2(F)
  Add Constant8/1 -> Add Constant9/1, Subsystem9/1(index)
  [Ff] -> Subsystem19/2(F)
  [Ff] -> Subsystem1/2(F)
  Subsystem19/1(Out1) -> Add/19
  Subsystem17/1(Out1) -> Add/18
  [Ff] -> Subsystem20/2(F)
  Add Constant10/1 -> Add Constant11/1, Subsystem10/1(index)
  Add Constant16/1 -> Add Constant17/1, Subsystem17/1(index)
  Subsystem6/1(Out1) -> Add/1
  Subsystem10/1(Out1) -> Add/12
  Subsystem5/1(Out1) -> Add/8
  [Ff] -> Subsystem10/2(F)
  [Ff] -> Subsystem15/2(F)
  Add Constant18/1 -> Subsystem18/1(index)
  Add Constant17/1 -> Add Constant18/1, Subsystem19/1(index)
  Subsystem7/1(Out1) -> Add/7
  Add Constant1/1 -> Add Constant2/1, Subsystem3/1(index)
  [Ff] -> Subsystem3/2(F)
  Subsystem9/1(Out1) -> Add/10
  Add Constant3/1 -> Add Constant4/1, Subsystem8/1(index)
  Add Constant/1 -> Add Constant1/1, Subsystem1/1(index)
  Subsystem1/1(Out1) -> Add/2
  Add Constant15/1 -> Add Constant16/1, Subsystem20/1(index)
  [Ff] -> Subsystem17/2(F)
  Subsystem8/1(Out1) -> Add/5
  Add Constant14/1 -> Add Constant15/1, Subsystem13/1(index)
  Add Constant11/1 -> Add Constant12/1, Subsystem16/1(index)
  [Ff] -> Subsystem13/2(F)
  Add Constant2/1 -> Add Constant3/1, Subsystem2/1(index)
  Add Constant9/1 -> Add Constant10/1, Subsystem11/1(index)
  Subsystem14/1(Out1) -> Add/9

== system Cruise_creator/Subsystem5/Subsystem1/Subsystem ==
  In1 : Inport
  In2 : Inport  Port=2
  Add : Sum  Inputs=++++++++++++++++++++
  Add Constant{,1..18} (19 alike) : Bias  Bias=1  SaturateOnIntegerOverflow=off
  Subsystem1 : Subsystem
  Subsystem10 : Subsystem
  Subsystem11 : Subsystem
  Subsystem12 : Subsystem
  Subsystem13 : Subsystem
  Subsystem14 : Subsystem
  Subsystem15 : Subsystem
  Subsystem16 : Subsystem
  Subsystem17 : Subsystem
  Subsystem18 : Subsystem
  Subsystem19 : Subsystem
  Subsystem2 : Subsystem
  Subsystem20 : Subsystem
  Subsystem3 : Subsystem
  Subsystem4 : Subsystem
  Subsystem5 : Subsystem
  Subsystem6 : Subsystem
  Subsystem7 : Subsystem
  Subsystem8 : Subsystem
  Subsystem9 : Subsystem
  Out1 : Outport
  wires:
  In2/1 -> [Ff]
  Add Constant18/1 -> Subsystem18/1(index)
  Add Constant17/1 -> Add Constant12/1, Subsystem20/1(index)
  Add Constant16/1 -> Add Constant17/1, Subsystem14/1(index)
  Subsystem2/1(Out1) -> Add/4
  Add Constant11/1 -> Add Constant14/1, Subsystem16/1(index)
  Subsystem6/1(Out1) -> Add/8
  Add Constant5/1 -> Add Constant6/1, Subsystem7/1(index)
  Add Constant9/1 -> Add Constant10/1, Subsystem11/1(index)
  [Ff] -> Subsystem13/2(F)
  [Ff] -> Subsystem12/2(F)
  Subsystem19/1(Out1) -> Add/19
  [Ff] -> Subsystem8/2(F)
  [Ff] -> Subsystem14/2(F)
  Add/1 -> Out1/1
  Subsystem7/1(Out1) -> Add/7
  Subsystem3/1(Out1) -> Add/3
  [Ff] -> Subsystem20/2(F)
  [Ff] -> Subsystem5/2(F)
  Subsystem14/1(Out1) -> Add/16
  [Ff] -> Subsystem16/2(F)
  [Ff] -> Subsystem1/2(F)
  Subsystem13/1(Out1) -> Add/9
  Subsystem17/1(Out1) -> Add/18
  [Ff] -> Subsystem4/2(F)
  [Ff] -> Subsystem18/2(F)
  Subsystem12/1(Out1) -> Add/14
  [Ff] -> Subsystem2/2(F)
  Add Constant2/1 -> Add Constant3/1, Subsystem2/1(index)
  Subsystem10/1(Out1) -> Add/12
  Add Constant1/1 -> Add Constant2/1, Subsystem3/1(index)
  [Ff] -> Subsystem9/2(F)
  Add Constant13/1 -> Add Constant18/1, Subsystem19/1(index)
  Subsystem1/1(Out1) -> Add/2
  [Ff] -> Subsystem15/2(F)
  Subsystem5/1(Out1) -> Add/1
  [Ff] -> Subsystem19/2(F)
  Subsystem11/1(Out1) -> Add/11
  [Ff] -> Subsystem11/2(F)
  Add Constant/1 -> Add Constant1/1, Subsystem1/1(index)
  Add Constant3/1 -> Add Constant4/1, Subsystem8/1(index)
  Add Constant12/1 -> Add Constant13/1, Subsystem17/1(index)
  Add Constant10/1 -> Add Constant11/1, Subsystem10/1(index)
  [Ff] -> Subsystem10/2(F)
  [Ff] -> Subsystem17/2(F)
  Subsystem20/1(Out1) -> Add/17
  In1/1 -> Subsystem5/1(index), Add Constant/1
  Subsystem16/1(Out1) -> Add/13
  Add Constant6/1 -> Add Constant7/1, Subsystem6/1(index)
  Subsystem4/1(Out1) -> Add/6
  Add Constant15/1 -> Add Constant16/1, Subsystem15/1(index)
  Subsystem18/1(Out1) -> Add/20
  Add Constant4/1 -> Add Constant5/1, Subsystem4/1(index)
  [Ff] -> Subsystem6/2(F)
  Subsystem9/1(Out1) -> Add/10
  Add Constant8/1 -> Add Constant9/1, Subsystem9/1(index)
  [Ff] -> Subsystem7/2(F)
  Subsystem8/1(Out1) -> Add/5
  Add Constant14/1 -> Add Constant15/1, Subsystem12/1(index)
  [Ff] -> Subsystem3/2(F)
  Subsystem15/1(Out1) -> Add/15
  Add Constant7/1 -> Add Constant8/1, Subsystem13/1(index)

== system Cruise_creator/Subsystem5/Subsystem1/Subsystem1 ==
  In1 : Inport
  In2 : Inport  Port=2
  Add : Sum  Inputs=++++++++++++++++++++
  Add Constant{,1..18} (19 alike) : Bias  Bias=1  SaturateOnIntegerOverflow=off
  Subsystem1 : Subsystem
  Subsystem10 : Subsystem
  Subsystem11 : Subsystem
  Subsystem12 : Subsystem
  Subsystem13 : Subsystem
  Subsystem14 : Subsystem
  Subsystem15 : Subsystem
  Subsystem16 : Subsystem
  Subsystem17 : Subsystem
  Subsystem18 : Subsystem
  Subsystem19 : Subsystem
  Subsystem2 : Subsystem
  Subsystem20 : Subsystem
  Subsystem3 : Subsystem
  Subsystem4 : Subsystem
  Subsystem5 : Subsystem
  Subsystem6 : Subsystem
  Subsystem7 : Subsystem
  Subsystem8 : Subsystem
  Subsystem9 : Subsystem
  Out1 : Outport
  wires:
  Add Constant7/1 -> Subsystem13/1(index), Add Constant8/1
  Subsystem15/1(Out1) -> Add/15
  [Ff] -> Subsystem3/2(F)
  Add Constant14/1 -> Subsystem12/1(index), Add Constant15/1
  Subsystem8/1(Out1) -> Add/5
  [Ff] -> Subsystem7/2(F)
  Add Constant8/1 -> Subsystem9/1(index), Add Constant9/1
  Subsystem9/1(Out1) -> Add/10
  [Ff] -> Subsystem6/2(F)
  Add Constant4/1 -> Subsystem4/1(index), Add Constant5/1
  Subsystem18/1(Out1) -> Add/20
  Add Constant15/1 -> Subsystem15/1(index), Add Constant16/1
  Subsystem4/1(Out1) -> Add/6
  Add Constant6/1 -> Subsystem6/1(index), Add Constant7/1
  Subsystem16/1(Out1) -> Add/13
  In1/1 -> Add Constant/1, Subsystem5/1(index)
  Subsystem20/1(Out1) -> Add/17
  [Ff] -> Subsystem17/2(F)
  [Ff] -> Subsystem10/2(F)
  Add Constant10/1 -> Subsystem10/1(index), Add Constant11/1
  Add Constant12/1 -> Subsystem17/1(index), Add Constant13/1
  Add Constant3/1 -> Subsystem8/1(index), Add Constant4/1
  Add Constant/1 -> Subsystem1/1(index), Add Constant1/1
  [Ff] -> Subsystem11/2(F)
  Subsystem11/1(Out1) -> Add/11
  [Ff] -> Subsystem19/2(F)
  Subsystem5/1(Out1) -> Add/1
  [Ff] -> Subsystem15/2(F)
  Subsystem1/1(Out1) -> Add/2
  Add Constant13/1 -> Subsystem19/1(index), Add Constant18/1
  [Ff] -> Subsystem9/2(F)
  Add Constant1/1 -> Subsystem3/1(index), Add Constant2/1
  Subsystem10/1(Out1) -> Add/12
  Add Constant2/1 -> Subsystem2/1(index), Add Constant3/1
  [Ff] -> Subsystem2/2(F)
  Subsystem12/1(Out1) -> Add/14
  [Ff] -> Subsystem18/2(F)
  [Ff] -> Subsystem4/2(F)
  Subsystem17/1(Out1) -> Add/18
  Subsystem13/1(Out1) -> Add/9
  [Ff] -> Subsystem1/2(F)
  [Ff] -> Subsystem16/2(F)
  Subsystem14/1(Out1) -> Add/16
  [Ff] -> Subsystem5/2(F)
  [Ff] -> Subsystem20/2(F)
  Subsystem3/1(Out1) -> Add/3
  Subsystem7/1(Out1) -> Add/7
  Add/1 -> Out1/1
  [Ff] -> Subsystem14/2(F)
  [Ff] -> Subsystem8/2(F)
  Subsystem19/1(Out1) -> Add/19
  [Ff] -> Subsystem12/2(F)
  [Ff] -> Subsystem13/2(F)
  Add Constant9/1 -> Subsystem11/1(index), Add Constant10/1
  Add Constant5/1 -> Subsystem7/1(index), Add Constant6/1
  Subsystem6/1(Out1) -> Add/8
  Add Constant11/1 -> Subsystem16/1(index), Add Constant14/1
  Subsystem2/1(Out1) -> Add/4
  Add Constant16/1 -> Subsystem14/1(index), Add Constant17/1
  Add Constant17/1 -> Subsystem20/1(index), Add Constant12/1
  Add Constant18/1 -> Subsystem18/1(index)
  In2/1 -> [Ff]

== system Cruise_creator/Subsystem5/Subsystem1/Subsystem2 ==
  In1 : Inport
  In2 : Inport  Port=2
  Add : Sum  Inputs=++++++++++++++++++++
  Add Constant{,1..18} (19 alike) : Bias  Bias=1  SaturateOnIntegerOverflow=off
  Subsystem1 : Subsystem
  Subsystem10 : Subsystem
  Subsystem11 : Subsystem
  Subsystem12 : Subsystem
  Subsystem13 : Subsystem
  Subsystem14 : Subsystem
  Subsystem15 : Subsystem
  Subsystem16 : Subsystem
  Subsystem17 : Subsystem
  Subsystem18 : Subsystem
  Subsystem19 : Subsystem
  Subsystem2 : Subsystem
  Subsystem20 : Subsystem
  Subsystem3 : Subsystem
  Subsystem4 : Subsystem
  Subsystem5 : Subsystem
  Subsystem6 : Subsystem
  Subsystem7 : Subsystem
  Subsystem8 : Subsystem
  Subsystem9 : Subsystem
  Out1 : Outport
  wires:
  In2/1 -> [Ff]
  Add Constant18/1 -> Subsystem18/1(index)
  Add Constant17/1 -> Add Constant12/1, Subsystem20/1(index)
  Add Constant16/1 -> Add Constant17/1, Subsystem14/1(index)
  Subsystem2/1(Out1) -> Add/4
  Add Constant11/1 -> Add Constant14/1, Subsystem16/1(index)
  Subsystem6/1(Out1) -> Add/8
  Add Constant5/1 -> Add Constant6/1, Subsystem7/1(index)
  Add Constant9/1 -> Add Constant10/1, Subsystem11/1(index)
  [Ff] -> Subsystem13/2(F)
  [Ff] -> Subsystem12/2(F)
  Subsystem19/1(Out1) -> Add/19
  [Ff] -> Subsystem8/2(F)
  [Ff] -> Subsystem14/2(F)
  Add/1 -> Out1/1
  Subsystem7/1(Out1) -> Add/7
  Subsystem3/1(Out1) -> Add/3
  [Ff] -> Subsystem20/2(F)
  [Ff] -> Subsystem5/2(F)
  Subsystem14/1(Out1) -> Add/16
  [Ff] -> Subsystem16/2(F)
  [Ff] -> Subsystem1/2(F)
  Subsystem13/1(Out1) -> Add/9
  Subsystem17/1(Out1) -> Add/18
  [Ff] -> Subsystem4/2(F)
  [Ff] -> Subsystem18/2(F)
  Subsystem12/1(Out1) -> Add/14
  [Ff] -> Subsystem2/2(F)
  Add Constant2/1 -> Add Constant3/1, Subsystem2/1(index)
  Subsystem10/1(Out1) -> Add/12
  Add Constant1/1 -> Add Constant2/1, Subsystem3/1(index)
  [Ff] -> Subsystem9/2(F)
  Add Constant13/1 -> Add Constant18/1, Subsystem19/1(index)
  Subsystem1/1(Out1) -> Add/2
  [Ff] -> Subsystem15/2(F)
  Subsystem5/1(Out1) -> Add/1
  [Ff] -> Subsystem19/2(F)
  Subsystem11/1(Out1) -> Add/11
  [Ff] -> Subsystem11/2(F)
  Add Constant/1 -> Add Constant1/1, Subsystem1/1(index)
  Add Constant3/1 -> Add Constant4/1, Subsystem8/1(index)
  Add Constant12/1 -> Add Constant13/1, Subsystem17/1(index)
  Add Constant10/1 -> Add Constant11/1, Subsystem10/1(index)
  [Ff] -> Subsystem10/2(F)
  [Ff] -> Subsystem17/2(F)
  Subsystem20/1(Out1) -> Add/17
  In1/1 -> Subsystem5/1(index), Add Constant/1
  Subsystem16/1(Out1) -> Add/13
  Add Constant6/1 -> Add Constant7/1, Subsystem6/1(index)
  Subsystem4/1(Out1) -> Add/6
  Add Constant15/1 -> Add Constant16/1, Subsystem15/1(index)
  Subsystem18/1(Out1) -> Add/20
  Add Constant4/1 -> Add Constant5/1, Subsystem4/1(index)
  [Ff] -> Subsystem6/2(F)
  Subsystem9/1(Out1) -> Add/10
  Add Constant8/1 -> Add Constant9/1, Subsystem9/1(index)
  [Ff] -> Subsystem7/2(F)
  Subsystem8/1(Out1) -> Add/5
  Add Constant14/1 -> Add Constant15/1, Subsystem12/1(index)
  [Ff] -> Subsystem3/2(F)
  Subsystem15/1(Out1) -> Add/15
  Add Constant7/1 -> Add Constant8/1, Subsystem13/1(index)

== system Cruise_creator/Subsystem5/Subsystem1/Subsystem3 ==
  In1 : Inport
  In2 : Inport  Port=2
  Add : Sum  Inputs=++++++++++++++++++++
  Add Constant{,1..18} (19 alike) : Bias  Bias=1  SaturateOnIntegerOverflow=off
  Subsystem1 : Subsystem
  Subsystem10 : Subsystem
  Subsystem11 : Subsystem
  Subsystem12 : Subsystem
  Subsystem13 : Subsystem
  Subsystem14 : Subsystem
  Subsystem15 : Subsystem
  Subsystem16 : Subsystem
  Subsystem17 : Subsystem
  Subsystem18 : Subsystem
  Subsystem19 : Subsystem
  Subsystem2 : Subsystem
  Subsystem20 : Subsystem
  Subsystem3 : Subsystem
  Subsystem4 : Subsystem
  Subsystem5 : Subsystem
  Subsystem6 : Subsystem
  Subsystem7 : Subsystem
  Subsystem8 : Subsystem
  Subsystem9 : Subsystem
  Out1 : Outport
  wires:
  Add Constant7/1 -> Subsystem13/1(index), Add Constant8/1
  Subsystem15/1(Out1) -> Add/15
  [Ff] -> Subsystem3/2(F)
  Add Constant14/1 -> Subsystem12/1(index), Add Constant15/1
  Subsystem8/1(Out1) -> Add/5
  [Ff] -> Subsystem7/2(F)
  Add Constant8/1 -> Subsystem9/1(index), Add Constant9/1
  Subsystem9/1(Out1) -> Add/10
  [Ff] -> Subsystem6/2(F)
  Add Constant4/1 -> Subsystem4/1(index), Add Constant5/1
  Subsystem18/1(Out1) -> Add/20
  Add Constant15/1 -> Subsystem15/1(index), Add Constant16/1
  Subsystem4/1(Out1) -> Add/6
  Add Constant6/1 -> Subsystem6/1(index), Add Constant7/1
  Subsystem16/1(Out1) -> Add/13
  In1/1 -> Add Constant/1, Subsystem5/1(index)
  Subsystem20/1(Out1) -> Add/17
  [Ff] -> Subsystem17/2(F)
  [Ff] -> Subsystem10/2(F)
  Add Constant10/1 -> Subsystem10/1(index), Add Constant11/1
  Add Constant12/1 -> Subsystem17/1(index), Add Constant13/1
  Add Constant3/1 -> Subsystem8/1(index), Add Constant4/1
  Add Constant/1 -> Subsystem1/1(index), Add Constant1/1
  [Ff] -> Subsystem11/2(F)
  Subsystem11/1(Out1) -> Add/11
  [Ff] -> Subsystem19/2(F)
  Subsystem5/1(Out1) -> Add/1
  [Ff] -> Subsystem15/2(F)
  Subsystem1/1(Out1) -> Add/2
  Add Constant13/1 -> Subsystem19/1(index), Add Constant18/1
  [Ff] -> Subsystem9/2(F)
  Add Constant1/1 -> Subsystem3/1(index), Add Constant2/1
  Subsystem10/1(Out1) -> Add/12
  Add Constant2/1 -> Subsystem2/1(index), Add Constant3/1
  [Ff] -> Subsystem2/2(F)
  Subsystem12/1(Out1) -> Add/14
  [Ff] -> Subsystem18/2(F)
  [Ff] -> Subsystem4/2(F)
  Subsystem17/1(Out1) -> Add/18
  Subsystem13/1(Out1) -> Add/9
  [Ff] -> Subsystem1/2(F)
  [Ff] -> Subsystem16/2(F)
  Subsystem14/1(Out1) -> Add/16
  [Ff] -> Subsystem5/2(F)
  [Ff] -> Subsystem20/2(F)
  Subsystem3/1(Out1) -> Add/3
  Subsystem7/1(Out1) -> Add/7
  Add/1 -> Out1/1
  [Ff] -> Subsystem14/2(F)
  [Ff] -> Subsystem8/2(F)
  Subsystem19/1(Out1) -> Add/19
  [Ff] -> Subsystem12/2(F)
  [Ff] -> Subsystem13/2(F)
  Add Constant9/1 -> Subsystem11/1(index), Add Constant10/1
  Add Constant5/1 -> Subsystem7/1(index), Add Constant6/1
  Subsystem6/1(Out1) -> Add/8
  Add Constant11/1 -> Subsystem16/1(index), Add Constant14/1
  Subsystem2/1(Out1) -> Add/4
  Add Constant16/1 -> Subsystem14/1(index), Add Constant17/1
  Add Constant17/1 -> Subsystem20/1(index), Add Constant12/1
  Add Constant18/1 -> Subsystem18/1(index)
  In2/1 -> [Ff]

== system Cruise_creator/Subsystem5/Subsystem1/Subsystem4 ==
  In1 : Inport
  In2 : Inport  Port=2
  Add : Sum  Inputs=++++++++++++++++++++
  Add Constant{,1..18} (19 alike) : Bias  Bias=1  SaturateOnIntegerOverflow=off
  Subsystem1 : Subsystem
  Subsystem10 : Subsystem
  Subsystem11 : Subsystem
  Subsystem12 : Subsystem
  Subsystem13 : Subsystem
  Subsystem14 : Subsystem
  Subsystem15 : Subsystem
  Subsystem16 : Subsystem
  Subsystem17 : Subsystem
  Subsystem18 : Subsystem
  Subsystem19 : Subsystem
  Subsystem2 : Subsystem
  Subsystem20 : Subsystem
  Subsystem3 : Subsystem
  Subsystem4 : Subsystem
  Subsystem5 : Subsystem
  Subsystem6 : Subsystem
  Subsystem7 : Subsystem
  Subsystem8 : Subsystem
  Subsystem9 : Subsystem
  Out1 : Outport
  wires:
  In2/1 -> [Ff]
  Add Constant18/1 -> Subsystem18/1(index)
  Add Constant17/1 -> Add Constant12/1, Subsystem20/1(index)
  Add Constant16/1 -> Add Constant17/1, Subsystem14/1(index)
  Subsystem2/1(Out1) -> Add/4
  Add Constant11/1 -> Add Constant14/1, Subsystem16/1(index)
  Subsystem6/1(Out1) -> Add/8
  Add Constant5/1 -> Add Constant6/1, Subsystem7/1(index)
  Add Constant9/1 -> Add Constant10/1, Subsystem11/1(index)
  [Ff] -> Subsystem13/2(F)
  [Ff] -> Subsystem12/2(F)
  Subsystem19/1(Out1) -> Add/19
  [Ff] -> Subsystem8/2(F)
  [Ff] -> Subsystem14/2(F)
  Add/1 -> Out1/1
  Subsystem7/1(Out1) -> Add/7
  Subsystem3/1(Out1) -> Add/3
  [Ff] -> Subsystem20/2(F)
  [Ff] -> Subsystem5/2(F)
  Subsystem14/1(Out1) -> Add/16
  [Ff] -> Subsystem16/2(F)
  [Ff] -> Subsystem1/2(F)
  Subsystem13/1(Out1) -> Add/9
  Subsystem17/1(Out1) -> Add/18
  [Ff] -> Subsystem4/2(F)
  [Ff] -> Subsystem18/2(F)
  Subsystem12/1(Out1) -> Add/14
  [Ff] -> Subsystem2/2(F)
  Add Constant2/1 -> Add Constant3/1, Subsystem2/1(index)
  Subsystem10/1(Out1) -> Add/12
  Add Constant1/1 -> Add Constant2/1, Subsystem3/1(index)
  [Ff] -> Subsystem9/2(F)
  Add Constant13/1 -> Add Constant18/1, Subsystem19/1(index)
  Subsystem1/1(Out1) -> Add/2
  [Ff] -> Subsystem15/2(F)
  Subsystem5/1(Out1) -> Add/1
  [Ff] -> Subsystem19/2(F)
  Subsystem11/1(Out1) -> Add/11
  [Ff] -> Subsystem11/2(F)
  Add Constant/1 -> Add Constant1/1, Subsystem1/1(index)
  Add Constant3/1 -> Add Constant4/1, Subsystem8/1(index)
  Add Constant12/1 -> Add Constant13/1, Subsystem17/1(index)
  Add Constant10/1 -> Add Constant11/1, Subsystem10/1(index)
  [Ff] -> Subsystem10/2(F)
  [Ff] -> Subsystem17/2(F)
  Subsystem20/1(Out1) -> Add/17
  In1/1 -> Subsystem5/1(index), Add Constant/1
  Subsystem16/1(Out1) -> Add/13
  Add Constant6/1 -> Add Constant7/1, Subsystem6/1(index)
  Subsystem4/1(Out1) -> Add/6
  Add Constant15/1 -> Add Constant16/1, Subsystem15/1(index)
  Subsystem18/1(Out1) -> Add/20
  Add Constant4/1 -> Add Constant5/1, Subsystem4/1(index)
  [Ff] -> Subsystem6/2(F)
  Subsystem9/1(Out1) -> Add/10
  Add Constant8/1 -> Add Constant9/1, Subsystem9/1(index)
  [Ff] -> Subsystem7/2(F)
  Subsystem8/1(Out1) -> Add/5
  Add Constant14/1 -> Add Constant15/1, Subsystem12/1(index)
  [Ff] -> Subsystem3/2(F)
  Subsystem15/1(Out1) -> Add/15
  Add Constant7/1 -> Add Constant8/1, Subsystem13/1(index)
